simulink model "TosAeb"  (R2021a)
settings: Solver=FixedStepDiscrete  StartTime=0.0  StopTime=inf  FixedStep=0.01  RelTol=1e-3
undefined symbols (not defined in the file or in its folder): ACCModSts, AccSts1, AEB_ObjectMovingOrientation, AEB_ObjectMovingState, CN_BTCActive, CN_CurrentGearR, CN_CurrentGearR_DHT, CN_PTCActive, CN_VDCActive, CN_VSC_EspOff, CN_VSC_FCW_AEB_Error, CN_VSC_FCW_AEB_NoDispay, CN_VSC_FCW_AEB_PerfmDgdtn, CN_VSC_FCW_AEB_TempUnAvlbl, CN_VSC_VehStandstill, CNaccelAvoidMax, CNaccelAvoidMin, CNbrakePedalPress, CNcomfortStrLeftAccelMax, CNcomfortStrLeftAccelMin, CNcomfortStrRightAccelMax, CNcomfortStrRightAccelMin, CNDrvDoorOpen, CNDrvModeEco, CNDrvModeNorm, CNDrvModeOther, CNDrvModeSport, CNDrvSBRreminder, CNNull, CNobjInvalidID, CNPassDoorOpen, CNPassSBRreminder, CNPEBSAccelNegInf, CNPEBSAccelNull, CNPEBSTimeInf, CNPEBSTimeNull, CNSTOPMAX, CNSTOPNULL, CNsubjWidth, CNSwtOFF, CNSwtON, CNTTCMAX, CNTTCNULL, CNVehConfigAEBCycNone, CNVehConfigAEBNone, CNVehConfigAEBPedNone, CNVehConfigFCWNone, CNVehConfigJANone, CollisionSeverity, FALSE, FCWSnvty, JACycObjCalcInfoDefaultParams, JAPedObjCalcInfoDefaultParams, JAVehObjCalcInfoDefaultParams, latCycObjCalcInfoDefaultParams, latPedObjCalcInfoDefaultParams, longObjCalcInfoDefaultParams, ModSts, movingStatus, ObjectType ... (+1112)
libraries: simulink x3031, AEBSFunctionLib x50, Ta_Library x33, AEBSStateMachineLib x23, Mylibrary x12, simulink_extras x7, ObjSelect_lib x3, dspstat3 x2
(part 72 of 102)

== system TosAeb/FcwAebJa_Step_sys/ALG_AEBSystem/ALG_AEBController/AEB_LatObjInfoCalcApp/LatPedObjCalc/Subsystem/latPedObjInfoCalc/ObjCrossFlgCal/PedPreCollisionCalcFunc/SubjBoundingBox/SubjLLCoordinate ==
  inputSignals : Inport
  Add2 : Sum  Inputs=+--  OutDataTypeStr=single
  Add3 : Sum  Inputs=+-+  OutDataTypeStr=single
  Bus Selector2 : BusSelector  OutputSignals=dxPreRefPoint,dxRearBumperToRearAxis,sincosCoef.cosCoef,subjWidth,sincosCoef.sinCoef
  Bus Selector3 : BusSelector  OutputSignals=dyPreRefPoint,dxRearBumperToRearAxis,sincosCoef.sinCoef,subjWidth,sincosCoef.cosCoef
  Gain2 : Gain  Gain=0.5  OutDataTypeStr=single
  Gain3 : Gain  Gain=0.5  OutDataTypeStr=single
  Product{4..7} (4 alike) : Product  OutDataTypeStr=single
  dxPreLL : Outport
  dyPreLL : Outport  Port=2
  wires:
  Gain3/1 -> Add3/3
  Bus Selector3/3 -> Product6/2  "<sinCoef>"
  Add3/1 -> dyPreLL/1
  Product7/1 -> Gain3/1
  Product6/1 -> Add3/2
  Product4/1 -> Add2/2
  Bus Selector3/5 -> Product7/2  "<cosCoef>"
  Product5/1 -> Gain2/1
  Gain2/1 -> Add2/3
  Add2/1 -> dxPreLL/1
  Bus Selector3/4 -> Product7/1  "<subjWidth>"
  Bus Selector3/2 -> Product6/1  "<dxRearBumperToRearAxis>"
  Bus Selector3/1 -> Add3/1  "<dyPreRefPoint>"
  inputSignals/1 -> Bus Selector3/1, Bus Selector2/1
  Bus Selector2/4 -> Product5/1  "<subjWidth>"
  Bus Selector2/2 -> Product4/1  "<dxRearBumperToRearAxis>"
  Bus Selector2/3 -> Product4/2  "<cosCoef>"
  Bus Selector2/1 -> Add2/1  "<dxPreRefPoint>"
  Bus Selector2/5 -> Product5/2  "<sinCoef>"

== system TosAeb/FcwAebJa_Step_sys/ALG_AEBSystem/ALG_AEBController/AEB_LatObjInfoCalcApp/LatPedObjCalc/Subsystem/latPedObjInfoCalc/ObjCrossFlgCal/PedPreCollisionCalcFunc/SubjBoundingBox/SubjLUCoordinate ==
  inputSignals : Inport
  Add : Sum  Inputs=++-  OutDataTypeStr=single
  Add1 : Sum  Inputs=+++  OutDataTypeStr=single
  Bus Selector : BusSelector  OutputSignals=dxPreRefPoint,dxFrontBumperToRearAxis,sincosCoef.cosCoef,subjWidth,sincosCoef.sinCoef
  Bus Selector1 : BusSelector  OutputSignals=dyPreRefPoint,dxFrontBumperToRearAxis,sincosCoef.sinCoef,subjWidth,sincosCoef.cosCoef
  Gain : Gain  Gain=0.5  OutDataTypeStr=single
  Gain1 : Gain  Gain=0.5  OutDataTypeStr=single
  Product{,1..3} (4 alike) : Product  OutDataTypeStr=single
  dxPreLU : Outport
  dyPreLU : Outport  Port=2
  wires:
  Bus Selector/4 -> Product1/1  "<subjWidth>"
  Product3/1 -> Gain1/1
  Add/1 -> dxPreLU/1
  Bus Selector1/1 -> Add1/1  "<dyPreRefPoint>"
  Gain1/1 -> Add1/3
  Bus Selector1/4 -> Product3/1  "<subjWidth>"
  Product2/1 -> Add1/2
  Bus Selector/3 -> Product/2  "<cosCoef>"
  Add1/1 -> dyPreLU/1
  Bus Selector/5 -> Product1/2  "<sinCoef>"
  Bus Selector/2 -> Product/1  "<dxFrontBumperToRearAxis>"
  Bus Selector1/2 -> Product2/1  "<dxFrontBumperToRearAxis>"
  inputSignals/1 -> Bus Selector1/1, Bus Selector/1
  Bus Selector1/5 -> Product3/2  "<cosCoef>"
  Bus Selector/1 -> Add/1  "<dxPreRefPoint>"
  Gain/1 -> Add/3
  Product1/1 -> Gain/1
  Product/1 -> Add/2
  Bus Selector1/3 -> Product2/2  "<sinCoef>"

== system TosAeb/FcwAebJa_Step_sys/ALG_AEBSystem/ALG_AEBController/AEB_LatObjInfoCalcApp/LatPedObjCalc/Subsystem/latPedObjInfoCalc/ObjCrossFlgCal/PedPreCollisionCalcFunc/SubjBoundingBox/SubjRLCoordinate ==
  inputSignals : Inport
  Add6 : Sum  Inputs=+-+  OutDataTypeStr=single
  Add7 : Sum  Inputs=+--  OutDataTypeStr=single
  Bus Selector6 : BusSelector  OutputSignals=dxPreRefPoint,dxRearBumperToRearAxis,sincosCoef.cosCoef,subjWidth,sincosCoef.sinCoef
  Bus Selector7 : BusSelector  OutputSignals=dyPreRefPoint,dxRearBumperToRearAxis,sincosCoef.sinCoef,subjWidth,sincosCoef.cosCoef
  Gain6 : Gain  Gain=0.5  OutDataTypeStr=single
  Gain7 : Gain  Gain=0.5  OutDataTypeStr=single
  Product{12..15} (4 alike) : Product  OutDataTypeStr=single
  dxPreRL : Outport
  dyPreRL : Outport  Port=2
  wires:
  Product12/1 -> Add6/2
  Gain6/1 -> Add6/3
  Product13/1 -> Gain6/1
  Add6/1 -> dxPreRL/1
  Bus Selector6/4 -> Product13/1  "<subjWidth>"
  Bus Selector6/5 -> Product13/2  "<sinCoef>"
  Bus Selector6/1 -> Add6/1  "<dxPreRefPoint>"
  Bus Selector6/2 -> Product12/1  "<dxRearBumperToRearAxis>"
  Bus Selector6/3 -> Product12/2  "<cosCoef>"
  inputSignals/1 -> Bus Selector7/1, Bus Selector6/1
  Add7/1 -> dyPreRL/1
  Product14/1 -> Add7/2
  Gain7/1 -> Add7/3
  Bus Selector7/1 -> Add7/1  "<dyPreRefPoint>"
  Bus Selector7/2 -> Product14/1  "<dxRearBumperToRearAxis>"
  Bus Selector7/4 -> Product15/1  "<subjWidth>"
  Product15/1 -> Gain7/1
  Bus Selector7/5 -> Product15/2  "<cosCoef>"
  Bus Selector7/3 -> Product14/2  "<sinCoef>"

== system TosAeb/FcwAebJa_Step_sys/ALG_AEBSystem/ALG_AEBController/AEB_LatObjInfoCalcApp/LatPedObjCalc/Subsystem/latPedObjInfoCalc/ObjCrossFlgCal/PedPreCollisionCalcFunc/SubjBoundingBox/SubjRUCoordinate ==
  inputSignals : Inport
  Add4 : Sum  Inputs=+++  OutDataTypeStr=single
  Add5 : Sum  Inputs=++-  OutDataTypeStr=single
  Bus Selector4 : BusSelector  OutputSignals=dxPreRefPoint,dxFrontBumperToRearAxis,sincosCoef.cosCoef,subjWidth,sincosCoef.sinCoef
  Bus Selector5 : BusSelector  OutputSignals=dyPreRefPoint,dxFrontBumperToRearAxis,sincosCoef.sinCoef,subjWidth,sincosCoef.cosCoef
  Gain4 : Gain  Gain=0.5  OutDataTypeStr=single
  Gain5 : Gain  Gain=0.5  OutDataTypeStr=single
  Product{8..11} (4 alike) : Product  OutDataTypeStr=single
  dxPreRU : Outport
  dyPreRU : Outport  Port=2
  wires:
  Bus Selector4/4 -> Product9/1  "<subjWidth>"
  Bus Selector4/5 -> Product9/2  "<sinCoef>"
  Bus Selector4/3 -> Product8/2  "<cosCoef>"
  Bus Selector4/1 -> Add4/1  "<dxPreRefPoint>"
  inputSignals/1 -> Bus Selector5/1, Bus Selector4/1
  Bus Selector4/2 -> Product8/1  "<dxFrontBumperToRearAxis>"
  Product9/1 -> Gain4/1
  Bus Selector5/4 -> Product11/1  "<subjWidth>"
  Product10/1 -> Add5/2
  Product11/1 -> Gain5/1
  Add5/1 -> dyPreRU/1
  Gain5/1 -> Add5/3
  Bus Selector5/2 -> Product10/1  "<dxFrontBumperToRearAxis>"
  Bus Selector5/3 -> Product10/2  "<sinCoef>"
  Bus Selector5/5 -> Product11/2  "<cosCoef>"
  Add4/1 -> dxPreRU/1
  Gain4/1 -> Add4/3
  Product8/1 -> Add4/2
  Bus Selector5/1 -> Add5/1  "<dyPreRefPoint>"

== system TosAeb/FcwAebJa_Step_sys/ALG_AEBSystem/ALG_AEBController/AEB_LongTgtInfoCalc/LongObjCalcInfoCalc/AEBS_LongCollisionAssesApp/LatCollisionAvoidCalc/AccelLatAvoidCalc4SO/accelLatAvoidLeftCalc/CalculateAccelSteeringLeft/CalculateAccelSteeringLeft  ==
  dxRelTargetObjCalc : Inport
  dyRelTargetObjCalc : Inport  Port=2
  subjVel : Inport  Port=3
  Function : TriggerPort  TriggerType=function-call  VariantControl=Variant  PortDimensions=1
  Add : Sum
  Add1 : Sum
  Divide : Product  Inputs=*/
  Divide1 : Product  Inputs=*/
  Gain : Gain  Gain=2
  Math Function{,1..3} (4 alike) : Math  Operator=square  SignedPower=on  SaturateOnIntegerOverflow=on
  P_LongCA_LatAvoidLatDistMinSO : Constant  Value=P_AEB_LongCA_LatAvoidLatDistMinSO
  Subtract : Sum  Inputs=+-
  accelLatAvoidLeft : Outport
  wires:
  Math Function/1 -> Add/1
  Math Function2/1 -> Add/2
  dyRelTargetObjCalc/1 -> Math Function2/1, Add1/2
  P_LongCA_LatAvoidLatDistMinSO/1 -> Add1/1, Math Function1/1
  Add/1 -> Subtract/1
  Math Function1/1 -> Subtract/2
  dxRelTargetObjCalc/1 -> Math Function/1
  Add1/1 -> Gain/1
  Subtract/1 -> Divide/1
  Gain/1 -> Divide/2
  Divide/1 -> Divide1/2
  Divide1/1 -> accelLatAvoidLeft/1
  subjVel/1 -> Math Function3/1
  Math Function3/1 -> Divide1/1

== system TosAeb/FcwAebJa_Step_sys/ALG_AEBSystem/ALG_AEBController/AEB_LongTgtInfoCalc/LongObjCalcInfoCalc/AEBS_LongCollisionAssesApp/LatCollisionAvoidCalc/AccelLatAvoidCalc4SO/accelLatAvoidLeftCalc/CalculateAccelSteeringLeft/tempFlgCalc ==
  dxRelTargetObjCalc : Inport
  dyRelTargetObjCalc : Inport  Port=2
  Add : Sum
  Math Function : Math  Operator=square  SignedPower=on  SaturateOnIntegerOverflow=on
  Math Function1 : Math  Operator=square  SignedPower=on  SaturateOnIntegerOverflow=on
  Math Function2 : Math  Operator=square  SignedPower=on  SaturateOnIntegerOverflow=on
  Relational Operator : RelationalOperator  Operator=<  OutDataTypeStr=boolean  RndMeth=Simplest
  dyMinStationary : Constant  Value=PcomfortStrLatDistMinSO
  tempFlg : Outport
  wires:
  Relational Operator/1 -> tempFlg/1
  Math Function/1 -> Add/1
  Math Function1/1 -> Relational Operator/1
  Add/1 -> Relational Operator/2
  Math Function2/1 -> Add/2
  dyMinStationary/1 -> Math Function1/1
  dyRelTargetObjCalc/1 -> Math Function2/1
  dxRelTargetObjCalc/1 -> Math Function/1

== system TosAeb/FcwAebJa_Step_sys/ALG_AEBSystem/ALG_AEBController/AEB_LongTgtInfoCalc/LongObjCalcInfoCalc/AEBS_LongCollisionAssesApp/LatCollisionAvoidCalc/AccelLatAvoidCalc4SO/accelLatAvoidRightCalc/CalculateAccelSteeringRight/CalculateAccelSteeringLeft  ==
  note: NOTE:
      | accelSteeringRight < 0
  dxRelTargetObjCalc : Inport
  dyRelTargetObjCalc : Inport  Port=2
  subjVel : Inport  Port=3
  Function : TriggerPort  TriggerType=function-call  VariantControl=Variant  PortDimensions=1
  Add : Sum
  Add1 : Sum  Inputs=+-
  Divide : Product  Inputs=*/
  Divide1 : Product  Inputs=*/
  Gain : Gain  Gain=2
  Gain1 : Gain  Gain=-1
  Math Function{,1..3} (4 alike) : Math  Operator=square  SignedPower=on  SaturateOnIntegerOverflow=on
  Subtract : Sum  Inputs=+-
  dyMin : Constant  Value=PcomfortStrLatDistMinSO
  accelLatAvoidRight : Outport
  wires:
  Gain1/1 -> accelLatAvoidRight/1
  Math Function/1 -> Add/1
  Math Function2/1 -> Add/2
  dyRelTargetObjCalc/1 -> Math Function2/1, Add1/2
  dyMin/1 -> Add1/1, Math Function1/1
  Add/1 -> Subtract/1
  Math Function1/1 -> Subtract/2
  dxRelTargetObjCalc/1 -> Math Function/1
  Add1/1 -> Gain/1
  Subtract/1 -> Divide/1
  Gain/1 -> Divide/2
  Divide/1 -> Divide1/2
  Divide1/1 -> Gain1/1
  subjVel/1 -> Math Function3/1
  Math Function3/1 -> Divide1/1

== system TosAeb/FcwAebJa_Step_sys/ALG_AEBSystem/ALG_AEBController/AEB_LongTgtInfoCalc/LongObjCalcInfoCalc/AEBS_LongCollisionAssesApp/LatCollisionAvoidCalc/AccelLatAvoidCalc4SO/accelLatAvoidRightCalc/CalculateAccelSteeringRight/tempFlgCalc ==
  dxRelTargetObjCalc : Inport
  dyRelTargetObjCalc : Inport  Port=2
  Add : Sum
  Math Function : Math  Operator=square  SignedPower=on  SaturateOnIntegerOverflow=on
  Math Function1 : Math  Operator=square  SignedPower=on  SaturateOnIntegerOverflow=on
  Math Function2 : Math  Operator=square  SignedPower=on  SaturateOnIntegerOverflow=on
  Relational Operator : RelationalOperator  Operator=<  OutDataTypeStr=boolean  RndMeth=Simplest
  dyMinStationary : Constant  Value=PcomfortStrLatDistMinSO
  tempFlg : Outport
  wires:
  Relational Operator/1 -> tempFlg/1
  Math Function/1 -> Add/1
  Math Function1/1 -> Relational Operator/1
  Add/1 -> Relational Operator/2
  Math Function2/1 -> Add/2
  dyMinStationary/1 -> Math Function1/1
  dyRelTargetObjCalc/1 -> Math Function2/1
  dxRelTargetObjCalc/1 -> Math Function/1

== system TosAeb/FcwAebJa_Step_sys/ALG_AEBSystem/ALG_AEBController/AEB_LongTgtInfoCalc/LongObjCalcInfoCalc/AEBS_LongCollisionAssesApp/LatCollisionAvoidCalc/AccelLatAvoidCalcMO/MovingObjLatCollisionAvoidCalc/Condition2/TTCIsZero ==
  TTC : Inport
  Compare To Constant : simulink/Logic and Bit Operations/Compare To Constant  relop=<  const=0.1  OutDataTypeStr=boolean
  TTCIsZeroFlg : Outport
  wires:
  Compare To Constant/1 -> TTCIsZeroFlg/1
  TTC/1 -> Compare To Constant/1

== system TosAeb/FcwAebJa_Step_sys/ALG_AEBSystem/ALG_AEBController/AEB_LongTgtInfoCalc/LongObjCalcInfoCalc/AEBS_LongCollisionAssesApp/LatCollisionAvoidCalc/AccelLatAvoidCalcMO/MovingObjLatCollisionAvoidCalc/Condition2/latAvoidAccelMO ==
  dy : Inport
  vy : Inport  Port=2
  TTC : Inport  Port=3
  Action Port : ActionPort  ActionPortLabel=else
  Bus Creator : BusCreator  Inputs=2
  Divide : Product  Inputs=*/
  Divide1 : Product  Inputs=*/
  Gain : Gain  Gain=-2
  Gain1 : Gain  Gain=-2
  Math Function : Math  Operator=square  SignedPower=on  SaturateOnIntegerOverflow=on
  Math Function1 : Math  Operator=square  SignedPower=on  SaturateOnIntegerOverflow=on
  P_LongCA_LatAvoidLatDistMinSO : Constant  Value=P_AEB_LongCA_LatAvoidLatDistMinSO
  P_LongCA_LatAvoidLatDistMinSO1 : Constant  Value=P_AEB_LongCA_LatAvoidLatDistMinSO
  Product : Product
  Product1 : Product
  Saturation : Saturate  UpperLimit=CNcomfortStrRightAccelMax  LowerLimit=CNcomfortStrRightAccelMin
      settings: AttributesFormatString=|
      | UpperLimit:%<UpperLimit>
      | LowerLimit:%<LowerLimit>
  Saturation1 : Saturate  UpperLimit=CNcomfortStrLeftAccelMax  LowerLimit=CNcomfortStrLeftAccelMin
      settings: AttributesFormatString=|
      | UpperLimit:%<UpperLimit>
      | LowerLimit:%<LowerLimit>
  Subtract : Sum  Inputs=+--
  Subtract1 : Sum  Inputs=---
  accelLatAvoid : Outport
  wires:
  Gain1/1 -> Divide/1
  Gain/1 -> Divide1/1
  Divide/1 -> Saturation/1  "comfortStrAccelRightUnLimited"
  Divide1/1 -> Saturation1/1  "comfortStrAccelLeftUnLimited"
  Bus Creator/1 -> accelLatAvoid/1
  vy/1 -> [vyRelTargetObjCalc], Product1/1
  TTC/1 -> Product/2, Math Function/1, Product1/2, Math Function1/1
  Product1/1 -> Subtract1/3
  P_LongCA_LatAvoidLatDistMinSO/1 -> Subtract1/1
  dy/1 -> [dyRelTargetObjCalc], Subtract1/2
  Math Function1/1 -> Divide1/2
  Subtract1/1 -> Gain/1
  Subtract/1 -> Gain1/1
  Math Function/1 -> Divide/2
  [dyRelTargetObjCalc] -> Subtract/2
  P_LongCA_LatAvoidLatDistMinSO1/1 -> Subtract/1
  Product/1 -> Subtract/3
  [vyRelTargetObjCalc] -> Product/1
  Saturation/1 -> Bus Creator/2  "accelLatAvoidRight"
  Saturation1/1 -> Bus Creator/1  "accelLatAvoidLeft"

== system TosAeb/FcwAebJa_Step_sys/ALG_AEBSystem/ALG_AEBController/AEB_LongTgtInfoCalc/LongObjCalcInfoCalc/AEBS_LongCollisionAssesApp/LatCollisionAvoidCalc/AccelLatAvoidCalcMO/MovingObjLatCollisionAvoidCalc/Condition2/latAvoidAccelMoMax ==
  Action Port : ActionPort  ActionPortLabel=if(u1)
  Bus Creator : BusCreator  Inputs=2
  Constant : Constant  Value=CNcomfortStrLeftAccelMax
  Constant1 : Constant  Value=CNcomfortStrRightAccelMin
  accelLatAvoid : Outport
  wires:
  Constant1/1 -> Bus Creator/2  "accelLatAvoidRight"
  Bus Creator/1 -> accelLatAvoid/1
  Constant/1 -> Bus Creator/1  "accelLatAvoidLeft"

== system TosAeb/FcwAebJa_Step_sys/ALG_AEBSystem/ALG_AEBController/AEB_LongTgtInfoCalc/LongObjCalcInfoCalc/AEBS_LongCollisionAssesApp/LongCollisionAvoidAssess/HighLvlBrkCalc/HighLvlBrkCalc4Brake/accelAvoidHighLvlBrkSituation1/accelAvoidLowLvlBrkCalc ==
  vx : Inport
  axAbsObj : Inport  Port=2
  vxAbsObj : Inport  Port=3
  subjVel : Inport  Port=4
  tObjStop : Inport  Port=5
  dxEgoBrake : Inport  Port=6
  AccelAvoidSituationJudge : Stateflow chart  TreatAsAtomicUnit=on
  Bus Creator : BusCreator  Inputs=6
  Bus Selector : BusSelector  OutputSignals=vx,axAbsObj
  Bus Selector1 : BusSelector  OutputSignals=dxEgoBrake,vx,tObjStop,subjVel,vxAbsObj,axAbsObj
  Bus Selector2 : BusSelector  OutputSignals=axAbsObj,vx,dxEgoBrake
  Bus Selector3 : BusSelector  OutputSignals=subjVel,vxAbsObj,axAbsObj,dxEgoBrake
  Merge : Merge  Inputs=4
  Situation1 : Subsystem  TreatAsAtomicUnit=on
  Situation2 : Subsystem  TreatAsAtomicUnit=on
  Situation3 : Subsystem  TreatAsAtomicUnit=on
  Situation4 : Subsystem  TreatAsAtomicUnit=on
  accelAvoidLowLvlBrkLimit : Subsystem
  accelAvoidLowLvlBrk : Outport
  wires:
  Bus Selector1/6 -> Situation1/6(axAbsObj)  "<axAbsObj>"
  Bus Selector1/5 -> Situation1/5(vxAbsObj)  "<vxAbsObj>"
  Bus Selector1/4 -> Situation1/4(subjVel)  "<subjVel>"
  Bus Selector1/3 -> Situation1/3(tObjStop)  "<tObjStop>"
  Bus Selector1/2 -> Situation1/2(vx)  "<vx>"
  Bus Selector1/1 -> Situation1/1(dxEgoBrake)  "<dxEgoBrake>"
  AccelAvoidSituationJudge/1(Situation1()) -> Situation1/Trigger
  Situation1/1(accelAvoidLowLvlBrk) -> Merge/1
  Situation2/1(accelAvoidLowLvlBrk) -> Merge/2
  Situation3/1(accelAvoidLowLvlBrk) -> Merge/3
  Situation4/1(accelAvoidLowLvlBrk) -> Merge/4
  AccelAvoidSituationJudge/2(Situation2()) -> [Situation2]
  AccelAvoidSituationJudge/3(Situation3()) -> [Situation3]
  AccelAvoidSituationJudge/4(Situation4()) -> [Situation4]
  [Situation2] -> Situation2/Trigger
  [Situation3] -> Situation3/Trigger
  [Situation4] -> Situation4/Trigger
  vxAbsObj/1 -> Bus Creator/3  "vxAbsObj"
  subjVel/1 -> Bus Creator/4  "subjVel"
  dxEgoBrake/1 -> Bus Creator/6  "dxEgoBrake"
  vx/1 -> Bus Creator/1  "vx"
  axAbsObj/1 -> Bus Creator/2  "axAbsObj"
  tObjStop/1 -> Bus Creator/5  "tObjStop"
  Bus Creator/1 -> Bus Selector3/1, Bus Selector2/1, Bus Selector1/1, Bus Selector/1
  Bus Selector/1 -> AccelAvoidSituationJudge/1(vx)  "<vx>"
  Bus Selector/2 -> AccelAvoidSituationJudge/2(axAbsObj)  "<axAbsObj>"
  Bus Selector2/3 -> Situation2/3(dxEgoBrake)  "<dxEgoBrake>"
  Bus Selector2/2 -> Situation2/2(vx)  "<vx>"
  Bus Selector2/1 -> Situation2/1(axAbsObj)  "<axAbsObj>"
  Bus Selector3/1 -> Situation3/1(subjVel)  "<subjVel>"
  Bus Selector3/2 -> Situation3/2(vxAbsObj)  "<vxAbsObj>"
  Bus Selector3/3 -> Situation3/3(axAbsObj)  "<axAbsObj>"
  Bus Selector3/4 -> Situation3/4(dxEgoBrake)  "<dxEgoBrake>"
  Merge/1 -> accelAvoidLowLvlBrkLimit/1(accelAvoidLowLvlBrkNoLimit)
  accelAvoidLowLvlBrkLimit/1(accelAvoidLowLvlBrk) -> accelAvoidLowLvlBrk/1

== system TosAeb/FcwAebJa_Step_sys/ALG_AEBSystem/ALG_AEBController/AEB_LongTgtInfoCalc/LongObjCalcInfoCalc/AEBS_LongCollisionAssesApp/LongCollisionAvoidAssess/HighLvlBrkCalc/HighLvlBrkCalc4Brake/accelAvoidHighLvlBrkSituation1/accelAvoidLowLvlBrkCalc2 ==
  vx : Inport
  axAbsObj : Inport  Port=2
  vxAbsObj : Inport  Port=3
  subjVel : Inport  Port=4
  tObjStop : Inport  Port=5
  dxEgoBrake : Inport  Port=6
  AccelAvoidSituationJudge : Stateflow chart  TreatAsAtomicUnit=on
  Bus Creator : BusCreator  Inputs=6
  Bus Selector : BusSelector  OutputSignals=vxRelTargetObjCalc,axAbsTargetObjCalc
  Bus Selector1 : BusSelector  OutputSignals=dxEgoBrake,vxRelTargetObjCalc,tObjStop,subjVel,vxAbsTargetObjCalc,axAbsTargetObjCalc
  Bus Selector2 : BusSelector  OutputSignals=axAbsTargetObjCalc,vxRelTargetObjCalc,dxEgoBrake
  Bus Selector3 : BusSelector  OutputSignals=subjVel,vxAbsTargetObjCalc,axAbsTargetObjCalc,dxEgoBrake
  Merge : Merge  Inputs=4
  Situation1 : Subsystem  TreatAsAtomicUnit=on
  Situation2 : Subsystem  TreatAsAtomicUnit=on
  Situation3 : Subsystem  TreatAsAtomicUnit=on
  Situation4 : Subsystem  TreatAsAtomicUnit=on
  accelAvoidLowLvlBrkLimit : Subsystem
  accelAvoidLowLvlBrk : Outport
  wires:
  accelAvoidLowLvlBrkLimit/1(accelAvoidLowLvlBrk) -> accelAvoidLowLvlBrk/1
  Merge/1 -> accelAvoidLowLvlBrkLimit/1(accelAvoidLowLvlBrkNoLimit)
  Bus Selector3/4 -> Situation3/4(dxEgoBrake)  "<dxEgoBrake>"
  Bus Selector3/3 -> Situation3/3(axAbsObj)  "<axAbsTargetObjCalc>"
  Bus Selector3/2 -> Situation3/2(vxAbsObj)  "<vxAbsTargetObjCalc>"
  Bus Selector3/1 -> Situation3/1(subjVel)  "<subjVel>"
  Bus Selector2/1 -> Situation2/1(axAbsObj)  "<axAbsTargetObjCalc>"
  Bus Selector2/2 -> Situation2/2(vx)  "<vxRelTargetObjCalc>"
  Bus Selector2/3 -> Situation2/3(dxEgoBrake)  "<dxEgoBrake>"
  Bus Selector/2 -> AccelAvoidSituationJudge/2(axAbsTargetObjCalc)  "<axAbsTargetObjCalc>"
  Bus Selector/1 -> AccelAvoidSituationJudge/1(vxRelTargetObjCalc)  "<vxRelTargetObjCalc>"
  Bus Creator/1 -> Bus Selector/1, Bus Selector1/1, Bus Selector2/1, Bus Selector3/1
  tObjStop/1 -> Bus Creator/5  "tObjStop"
  axAbsObj/1 -> Bus Creator/2  "axAbsTargetObjCalc"
  vx/1 -> Bus Creator/1  "vxRelTargetObjCalc"
  dxEgoBrake/1 -> Bus Creator/6  "dxEgoBrake"
  subjVel/1 -> Bus Creator/4  "subjVel"
  vxAbsObj/1 -> Bus Creator/3  "vxAbsTargetObjCalc"
  [Situation4] -> Situation4/Trigger
  [Situation3] -> Situation3/Trigger
  [Situation2] -> Situation2/Trigger
  AccelAvoidSituationJudge/4(Situation4()) -> [Situation4]
  AccelAvoidSituationJudge/3(Situation3()) -> [Situation3]
  AccelAvoidSituationJudge/2(Situation2()) -> [Situation2]
  Situation4/1(accelAvoidLowLvlBrk) -> Merge/4
  Situation3/1(accelAvoidLowLvlBrk) -> Merge/3
  Situation2/1(accelAvoidLowLvlBrk) -> Merge/2
  Situation1/1(accelAvoidLowLvlBrk) -> Merge/1
  AccelAvoidSituationJudge/1(Situation1()) -> Situation1/Trigger
  Bus Selector1/1 -> Situation1/1(dxEgoBrake)  "<dxEgoBrake>"
  Bus Selector1/2 -> Situation1/2(vx)  "<vxRelTargetObjCalc>"
  Bus Selector1/3 -> Situation1/3(tObjStop)  "<tObjStop>"
  Bus Selector1/4 -> Situation1/4(subjVel)  "<subjVel>"
  Bus Selector1/5 -> Situation1/5(vxAbsObj)  "<vxAbsTargetObjCalc>"
  Bus Selector1/6 -> Situation1/6(axAbsObj)  "<axAbsTargetObjCalc>"

== system TosAeb/FcwAebJa_Step_sys/ALG_AEBSystem/ALG_AEBController/AEB_LongTgtInfoCalc/LongObjCalcInfoCalc/AEBS_LongCollisionAssesApp/LongCollisionAvoidAssess/HighLvlBrkCalc/HighLvlBrkCalc4Brake/accelAvoidHighLvlBrkSituation2/CollisionJuge ==
  tReaction : Inport
  TTC : Inport  Port=2
  Relational Operator : RelationalOperator  Operator=>  OutDataTypeStr=boolean  RndMeth=Simplest
  collisonFlg : Outport
  wires:
  TTC/1 -> Relational Operator/2
  Relational Operator/1 -> collisonFlg/1
  tReaction/1 -> Relational Operator/1

== system TosAeb/FcwAebJa_Step_sys/ALG_AEBSystem/ALG_AEBController/AEB_LongTgtInfoCalc/LongObjCalcInfoCalc/AEBS_LongCollisionAssesApp/LongCollisionAvoidAssess/HighLvlBrkCalc/HighLvlBrkCalc4Brake/accelAvoidHighLvlBrkSituation2/CollisonOcurred ==
  Action Port : ActionPort  ActionPortLabel=if(u1)
  Constant : Constant  Value=CNPEBSAccelNegInf
  accelAvoidHighLvlBrk : Outport
  wires:
  Constant/1 -> accelAvoidHighLvlBrk/1  "CNPEBSAccelNegInf"

== system TosAeb/FcwAebJa_Step_sys/ALG_AEBSystem/ALG_AEBController/AEB_LongTgtInfoCalc/LongObjCalcInfoCalc/AEBS_LongCollisionAssesApp/LongCollisionAvoidAssess/HighLvlBrkCalc/HighLvlBrkCalc4Brake/accelAvoidHighLvlBrkSituation2/accelAvoidHighLvlBrkCalc ==
  dxFrontBumper : Inport
  vx : Inport  Port=2
  tReaction : Inport  Port=3
  ax : Inport  Port=4
  subjLongAccel : Inport  Port=5
  subjVel : Inport  Port=6
  axAbsObj : Inport  Port=7
  Action Port : ActionPort  ActionPortLabel=else
  If : If  IfExpression=u1 <= 0
  If Action Subsystem : Subsystem  TreatAsAtomicUnit=on
  If Action Subsystem1 : Subsystem  TreatAsAtomicUnit=on
  Merge : Merge
  MovingSateCalcAfterReactionTime : Subsystem
  tObjStopCalc : Subsystem  TreatAsAtomicUnit=on
  accelAvoidHighLvlBrk : Outport
  wires:
  tObjStopCalc/1(tStop) -> If Action Subsystem1/5(tObjStop)
  Merge/1 -> accelAvoidHighLvlBrk/1
  vx/1 -> MovingSateCalcAfterReactionTime/2(vx)
  tReaction/1 -> MovingSateCalcAfterReactionTime/3(tReaction)
  ax/1 -> MovingSateCalcAfterReactionTime/4(ax)
  subjVel/1 -> MovingSateCalcAfterReactionTime/6(subjVel)
  subjLongAccel/1 -> MovingSateCalcAfterReactionTime/5(subjLongAccel)
  MovingSateCalcAfterReactionTime/1(dxFrontBumperPredict) -> [dxRelTargetPredict], If/1
  If/1 -> If Action Subsystem/Action
  If/2 -> If Action Subsystem1/Action
  If Action Subsystem1/1(accelAvoidHighLvlBrk) -> Merge/2
  If Action Subsystem/1(accelAvoidHighLvlBrk) -> Merge/1
  MovingSateCalcAfterReactionTime/3(vxAbsObjPredict) -> tObjStopCalc/2(velRel), If Action Subsystem1/3(vxAbsObj)
  axAbsObj/1 -> tObjStopCalc/1(accelRel), If Action Subsystem1/2(axAbsObj)
  MovingSateCalcAfterReactionTime/2(vxPredict) -> If Action Subsystem1/1(vx)
  MovingSateCalcAfterReactionTime/4(subjVelPredict) -> [subjVelPredict]
  [subjVelPredict] -> If Action Subsystem1/4(subjVel)
  [dxRelTargetPredict] -> If Action Subsystem1/6(dxEgoBrake)
  dxFrontBumper/1 -> MovingSateCalcAfterReactionTime/1(dxFrontBumper)

== system TosAeb/FcwAebJa_Step_sys/ALG_AEBSystem/ALG_AEBController/AEB_LongTgtInfoCalc/LongObjCalcInfoCalc/AEBS_LongCollisionAssesApp/LongCollisionAvoidAssess/HighLvlBrkCalc/HighLvlBrkCalc4Brake/accelAvoidHighLvlBrkSituation3/CollisionJuge ==
  tReaction : Inport
  TTC : Inport  Port=2
  Relational Operator : RelationalOperator  Operator=>  OutDataTypeStr=boolean  RndMeth=Simplest
  collisonFlg : Outport
  wires:
  TTC/1 -> Relational Operator/2
  Relational Operator/1 -> collisonFlg/1
  tReaction/1 -> Relational Operator/1

== system TosAeb/FcwAebJa_Step_sys/ALG_AEBSystem/ALG_AEBController/AEB_LongTgtInfoCalc/LongObjCalcInfoCalc/AEBS_LongCollisionAssesApp/LongCollisionAvoidAssess/HighLvlBrkCalc/HighLvlBrkCalc4Brake/accelAvoidHighLvlBrkSituation3/If Action Subsystem ==
  Action Port : ActionPort  ActionPortLabel=if(u1)
  Constant : Constant  Value=CNaccelAvoidMin
  accelAvoidHighLvlBrk : Outport
  wires:
  Constant/1 -> accelAvoidHighLvlBrk/1  "CNPEBSAccelNegInf"

== system TosAeb/FcwAebJa_Step_sys/ALG_AEBSystem/ALG_AEBController/AEB_LongTgtInfoCalc/LongObjCalcInfoCalc/AEBS_LongCollisionAssesApp/LongCollisionAvoidAssess/HighLvlBrkCalc/HighLvlBrkCalc4Brake/accelAvoidHighLvlBrkSituation3/If Action Subsystem1 ==
  subjLongAccel : Inport
  tReaction : Inport  Port=2
  subjVel : Inport  Port=3
  axAbsObj : Inport  Port=4
  vxAbsObj : Inport  Port=5
  dxFrontBumper : Inport  Port=6
  Action Port : ActionPort  ActionPortLabel=else
  CollisionOcurredCase : Subsystem  TreatAsAtomicUnit=on
  DistReactionCalc : Subsystem
  If : If  IfExpression=u1
  Merge : Merge
  accelAvoidHighLvlBrkCalc : Subsystem  TreatAsAtomicUnit=on
  accelAvoidHighLvlBrk : Outport
  wires:
  subjLongAccel/1 -> [subjAccel], DistReactionCalc/1(subjLongAccel)
  tReaction/1 -> [tReaction], DistReactionCalc/2(tReaction)
  subjVel/1 -> [subjVel], DistReactionCalc/3(subjVel)
  axAbsObj/1 -> DistReactionCalc/4(axAbsObj)
  vxAbsObj/1 -> DistReactionCalc/5(vxAbsObj)
  Merge/1 -> accelAvoidHighLvlBrk/1
  dxFrontBumper/1 -> DistReactionCalc/6(dxFrontBumper)
  DistReactionCalc/1(collisionOcurredFlag) -> If/1
  If/1 -> CollisionOcurredCase/Action
  If/2 -> accelAvoidHighLvlBrkCalc/Action
  CollisionOcurredCase/1(accelAvoidHighLvlBrk) -> Merge/1
  DistReactionCalc/2(dxRelTargetPredict) -> accelAvoidHighLvlBrkCalc/4(dxEgoBrake)
  [subjAccel] -> accelAvoidHighLvlBrkCalc/1(subjLongAccel)
  [tReaction] -> accelAvoidHighLvlBrkCalc/2(tReaction)
  [subjVel] -> accelAvoidHighLvlBrkCalc/3(subjVel)
  accelAvoidHighLvlBrkCalc/1(accelAvoidHighLvlBrk) -> Merge/2

== system TosAeb/FcwAebJa_Step_sys/ALG_AEBSystem/ALG_AEBController/AEB_LongTgtInfoCalc/LongObjCalcInfoCalc/AEBS_LongCollisionAssesApp/LongCollisionAvoidAssess/HighLvlBrkCalc/HighLvlBrkCalc4Brake/accelAvoidHighLvlBrkSituation4/accelAvoidHighLvlBrkCalc ==
  tReaction : Inport
  TTC : Inport  Port=2
  Constant : Constant  Value=CNaccelAvoidMax
  Constant1 : Constant  Value=CNaccelAvoidMin
  Relational Operator : RelationalOperator  Operator=<=  OutDataTypeStr=boolean  RndMeth=Simplest
  Switch : Switch  Criteria=u2 ~= 0
  accelAvoidHighLvlBrk : Outport
  wires:
  tReaction/1 -> Relational Operator/1
  Relational Operator/1 -> Switch/2
  TTC/1 -> Relational Operator/2
  Constant/1 -> Switch/1
  Constant1/1 -> Switch/3
  Switch/1 -> accelAvoidHighLvlBrk/1

== system TosAeb/FcwAebJa_Step_sys/ALG_AEBSystem/ALG_AEBController/AEB_LongTgtInfoCalc/LongObjCalcInfoCalc/AEBS_LongCollisionAssesApp/LongCollisionAvoidAssess/HighLvlBrkCalc/HighLvlBrkCalc4Brake/accelAvoidHighLvlBrkSituation5/If Action Subsystem ==
  tReaction : Inport
  TTC : Inport  Port=2
  Action Port : ActionPort  ActionPortLabel=if(u1 <= u2)
  Constant : Constant  Value=CNaccelAvoidMax
  Constant1 : Constant  Value=CNaccelAvoidMin
  Relational Operator : RelationalOperator  Operator=<=  OutDataTypeStr=boolean  RndMeth=Simplest
  Switch : Switch  Criteria=u2 > Threshold
  accelAvoidHighLvlBrk : Outport
  wires:
  tReaction/1 -> Relational Operator/1
  TTC/1 -> Relational Operator/2
  Relational Operator/1 -> Switch/2
  Constant/1 -> Switch/1
  Constant1/1 -> Switch/3
  Switch/1 -> accelAvoidHighLvlBrk/1

== system TosAeb/FcwAebJa_Step_sys/ALG_AEBSystem/ALG_AEBController/AEB_LongTgtInfoCalc/LongObjCalcInfoCalc/AEBS_LongCollisionAssesApp/LongCollisionAvoidAssess/HighLvlBrkCalc/HighLvlBrkCalc4Brake/accelAvoidHighLvlBrkSituation5/If Action Subsystem1 ==
  tReaction : Inport
  TTC : Inport  Port=2
  subjLongAccel : Inport  Port=3
  tEgoStop : Inport  Port=4
  subjVel : Inport  Port=5
  axAbsObj : Inport  Port=6
  dxFrontBumper : Inport  Port=7
  vxAbsObj : Inport  Port=8
  Action Port : ActionPort  ActionPortLabel=else
  CollisionJuge : Subsystem
  If : If  IfExpression=u1
  If Action Subsystem : Subsystem  TreatAsAtomicUnit=on
  If Action Subsystem1 : Subsystem  TreatAsAtomicUnit=on
  Merge : Merge
  accelAvoidHighLvlBrk : Outport
  wires:
  tEgoStop/1 -> If Action Subsystem1/2(tEgoStop)
  CollisionJuge/1(collisonFlg) -> If/1
  TTC/1 -> CollisionJuge/2(TTC)
  If/1 -> If Action Subsystem/Action
  If/2 -> If Action Subsystem1/Action
  If Action Subsystem/1(accelAvoidHighLvlBrk) -> Merge/1
  If Action Subsystem1/1(accelAvoidHighLvlBrk) -> Merge/2
  Merge/1 -> accelAvoidHighLvlBrk/1
  tReaction/1 -> CollisionJuge/1(tReaction)
  subjLongAccel/1 -> If Action Subsystem1/1(subjLongAccel)
  subjVel/1 -> If Action Subsystem1/3(subjVel)
  axAbsObj/1 -> If Action Subsystem1/4(axAbsObj)
  vxAbsObj/1 -> If Action Subsystem1/5(vxAbsObj)
  dxFrontBumper/1 -> If Action Subsystem1/6(dxFrontBumper)

== system TosAeb/FcwAebJa_Step_sys/ALG_AEBSystem/ALG_AEBController/AEB_LongTgtInfoCalc/LongObjCalcInfoCalc/AEBS_LongCollisionAssesApp/LongCollisionAvoidAssess/HighLvlBrkCalc/HighLvlBrkCalc4Warning/accelAvoidHighLvlBrkSituation1/accelAvoidLowLvlBrkCalc ==
  vx : Inport
  axAbsObj : Inport  Port=2
  vxAbsObj : Inport  Port=3
  subjVel : Inport  Port=4
  tObjStop : Inport  Port=5
  dxEgoBrake : Inport  Port=6
  AccelAvoidSituationJudge : Stateflow chart  TreatAsAtomicUnit=on
  Bus Creator : BusCreator  Inputs=6
  Bus Selector : BusSelector  OutputSignals=vx,axAbsObj
  Bus Selector1 : BusSelector  OutputSignals=dxEgoBrake,vx,tObjStop,subjVel,vxAbsObj,axAbsObj
  Bus Selector2 : BusSelector  OutputSignals=axAbsObj,vx,dxEgoBrake
  Bus Selector3 : BusSelector  OutputSignals=subjVel,vxAbsObj,axAbsObj,dxEgoBrake
  Merge : Merge  Inputs=4
  Situation1 : Subsystem  TreatAsAtomicUnit=on
  Situation2 : Subsystem  TreatAsAtomicUnit=on
  Situation3 : Subsystem  TreatAsAtomicUnit=on
  Situation4 : Subsystem  TreatAsAtomicUnit=on
  accelAvoidLowLvlBrkLimit : Subsystem
  accelAvoidLowLvlBrk : Outport
  wires:
  accelAvoidLowLvlBrkLimit/1(accelAvoidLowLvlBrk) -> accelAvoidLowLvlBrk/1
  Merge/1 -> accelAvoidLowLvlBrkLimit/1(accelAvoidLowLvlBrkNoLimit)
  Bus Selector3/4 -> Situation3/4(dxEgoBrake)  "<dxEgoBrake>"
  Bus Selector3/3 -> Situation3/3(axAbsObj)  "<axAbsObj>"
  Bus Selector3/2 -> Situation3/2(vxAbsObj)  "<vxAbsObj>"
  Bus Selector3/1 -> Situation3/1(subjVel)  "<subjVel>"
  Bus Selector2/1 -> Situation2/1(axAbsObj)  "<axAbsObj>"
  Bus Selector2/2 -> Situation2/2(vx)  "<vx>"
  Bus Selector2/3 -> Situation2/3(dxEgoBrake)  "<dxEgoBrake>"
  Bus Selector/2 -> AccelAvoidSituationJudge/2(axAbsObj)  "<axAbsObj>"
  Bus Selector/1 -> AccelAvoidSituationJudge/1(vx)  "<vx>"
  Bus Creator/1 -> Bus Selector/1, Bus Selector1/1, Bus Selector2/1, Bus Selector3/1
  tObjStop/1 -> Bus Creator/5  "tObjStop"
  axAbsObj/1 -> Bus Creator/2  "axAbsObj"
  vx/1 -> Bus Creator/1  "vx"
  dxEgoBrake/1 -> Bus Creator/6  "dxEgoBrake"
  subjVel/1 -> Bus Creator/4  "subjVel"
  vxAbsObj/1 -> Bus Creator/3  "vxAbsObj"
  [Situation4] -> Situation4/Trigger
  [Situation3] -> Situation3/Trigger
  [Situation2] -> Situation2/Trigger
  AccelAvoidSituationJudge/4(Situation4()) -> [Situation4]
  AccelAvoidSituationJudge/3(Situation3()) -> [Situation3]
  AccelAvoidSituationJudge/2(Situation2()) -> [Situation2]
  Situation4/1(accelAvoidLowLvlBrk) -> Merge/4
  Situation3/1(accelAvoidLowLvlBrk) -> Merge/3
  Situation2/1(accelAvoidLowLvlBrk) -> Merge/2
  Situation1/1(accelAvoidLowLvlBrk) -> Merge/1
  AccelAvoidSituationJudge/1(Situation1()) -> Situation1/Trigger
  Bus Selector1/1 -> Situation1/1(dxEgoBrake)  "<dxEgoBrake>"
  Bus Selector1/2 -> Situation1/2(vx)  "<vx>"
  Bus Selector1/3 -> Situation1/3(tObjStop)  "<tObjStop>"
  Bus Selector1/4 -> Situation1/4(subjVel)  "<subjVel>"
  Bus Selector1/5 -> Situation1/5(vxAbsObj)  "<vxAbsObj>"
  Bus Selector1/6 -> Situation1/6(axAbsObj)  "<axAbsObj>"

== system TosAeb/FcwAebJa_Step_sys/ALG_AEBSystem/ALG_AEBController/AEB_LongTgtInfoCalc/LongObjCalcInfoCalc/AEBS_LongCollisionAssesApp/LongCollisionAvoidAssess/HighLvlBrkCalc/HighLvlBrkCalc4Warning/accelAvoidHighLvlBrkSituation1/accelAvoidLowLvlBrkCalc2 ==
  vx : Inport
  axAbsObj : Inport  Port=2
  vxAbsObj : Inport  Port=3
  subjVel : Inport  Port=4
  tObjStop : Inport  Port=5
  dxEgoBrake : Inport  Port=6
  AccelAvoidSituationJudge : Stateflow chart  TreatAsAtomicUnit=on
  Bus Creator : BusCreator  Inputs=6
  Bus Selector : BusSelector  OutputSignals=vxRelTargetObjCalc,axAbsTargetObjCalc
  Bus Selector1 : BusSelector  OutputSignals=dxEgoBrake,vxRelTargetObjCalc,tObjStop,subjVel,vxAbsTargetObjCalc,axAbsTargetObjCalc
  Bus Selector2 : BusSelector  OutputSignals=axAbsTargetObjCalc,vxRelTargetObjCalc,dxEgoBrake
  Bus Selector3 : BusSelector  OutputSignals=subjVel,vxAbsTargetObjCalc,axAbsTargetObjCalc,dxEgoBrake
  Merge : Merge  Inputs=4
  Situation1 : Subsystem  TreatAsAtomicUnit=on
  Situation2 : Subsystem  TreatAsAtomicUnit=on
  Situation3 : Subsystem  TreatAsAtomicUnit=on
  Situation4 : Subsystem  TreatAsAtomicUnit=on
  accelAvoidLowLvlBrkLimit : Subsystem
  accelAvoidLowLvlBrk : Outport
  wires:
  Bus Selector1/6 -> Situation1/6(axAbsObj)  "<axAbsTargetObjCalc>"
  Bus Selector1/5 -> Situation1/5(vxAbsObj)  "<vxAbsTargetObjCalc>"
  Bus Selector1/4 -> Situation1/4(subjVel)  "<subjVel>"
  Bus Selector1/3 -> Situation1/3(tObjStop)  "<tObjStop>"
  Bus Selector1/2 -> Situation1/2(vx)  "<vxRelTargetObjCalc>"
  Bus Selector1/1 -> Situation1/1(dxEgoBrake)  "<dxEgoBrake>"
  AccelAvoidSituationJudge/1(Situation1()) -> Situation1/Trigger
  Situation1/1(accelAvoidLowLvlBrk) -> Merge/1
  Situation2/1(accelAvoidLowLvlBrk) -> Merge/2
  Situation3/1(accelAvoidLowLvlBrk) -> Merge/3
  Situation4/1(accelAvoidLowLvlBrk) -> Merge/4
  AccelAvoidSituationJudge/2(Situation2()) -> [Situation2]
  AccelAvoidSituationJudge/3(Situation3()) -> [Situation3]
  AccelAvoidSituationJudge/4(Situation4()) -> [Situation4]
  [Situation2] -> Situation2/Trigger
  [Situation3] -> Situation3/Trigger
  [Situation4] -> Situation4/Trigger
  vxAbsObj/1 -> Bus Creator/3  "vxAbsTargetObjCalc"
  subjVel/1 -> Bus Creator/4  "subjVel"
  dxEgoBrake/1 -> Bus Creator/6  "dxEgoBrake"
  vx/1 -> Bus Creator/1  "vxRelTargetObjCalc"
  axAbsObj/1 -> Bus Creator/2  "axAbsTargetObjCalc"
  tObjStop/1 -> Bus Creator/5  "tObjStop"
  Bus Creator/1 -> Bus Selector3/1, Bus Selector2/1, Bus Selector1/1, Bus Selector/1
  Bus Selector/1 -> AccelAvoidSituationJudge/1(vxRelTargetObjCalc)  "<vxRelTargetObjCalc>"
  Bus Selector/2 -> AccelAvoidSituationJudge/2(axAbsTargetObjCalc)  "<axAbsTargetObjCalc>"
  Bus Selector2/3 -> Situation2/3(dxEgoBrake)  "<dxEgoBrake>"
  Bus Selector2/2 -> Situation2/2(vx)  "<vxRelTargetObjCalc>"
  Bus Selector2/1 -> Situation2/1(axAbsObj)  "<axAbsTargetObjCalc>"
  Bus Selector3/1 -> Situation3/1(subjVel)  "<subjVel>"
  Bus Selector3/2 -> Situation3/2(vxAbsObj)  "<vxAbsTargetObjCalc>"
  Bus Selector3/3 -> Situation3/3(axAbsObj)  "<axAbsTargetObjCalc>"
  Bus Selector3/4 -> Situation3/4(dxEgoBrake)  "<dxEgoBrake>"
  Merge/1 -> accelAvoidLowLvlBrkLimit/1(accelAvoidLowLvlBrkNoLimit)
  accelAvoidLowLvlBrkLimit/1(accelAvoidLowLvlBrk) -> accelAvoidLowLvlBrk/1

== system TosAeb/FcwAebJa_Step_sys/ALG_AEBSystem/ALG_AEBController/AEB_LongTgtInfoCalc/LongObjCalcInfoCalc/AEBS_LongCollisionAssesApp/LongCollisionAvoidAssess/HighLvlBrkCalc/HighLvlBrkCalc4Warning/accelAvoidHighLvlBrkSituation2/CollisionJuge ==
  tReaction : Inport
  TTC : Inport  Port=2
  Relational Operator : RelationalOperator  Operator=>  OutDataTypeStr=boolean  RndMeth=Simplest
  collisonFlg : Outport
  wires:
  tReaction/1 -> Relational Operator/1
  Relational Operator/1 -> collisonFlg/1
  TTC/1 -> Relational Operator/2

== system TosAeb/FcwAebJa_Step_sys/ALG_AEBSystem/ALG_AEBController/AEB_LongTgtInfoCalc/LongObjCalcInfoCalc/AEBS_LongCollisionAssesApp/LongCollisionAvoidAssess/HighLvlBrkCalc/HighLvlBrkCalc4Warning/accelAvoidHighLvlBrkSituation2/CollisonOcurred ==
  Action Port : ActionPort  ActionPortLabel=if(u1)
  Constant : Constant  Value=CNPEBSAccelNegInf
  accelAvoidHighLvlBrk : Outport
  wires:
  Constant/1 -> accelAvoidHighLvlBrk/1  "CNPEBSAccelNegInf"

== system TosAeb/FcwAebJa_Step_sys/ALG_AEBSystem/ALG_AEBController/AEB_LongTgtInfoCalc/LongObjCalcInfoCalc/AEBS_LongCollisionAssesApp/LongCollisionAvoidAssess/HighLvlBrkCalc/HighLvlBrkCalc4Warning/accelAvoidHighLvlBrkSituation2/accelAvoidHighLvlBrkCalc ==
  dxFrontBumper : Inport
  vx : Inport  Port=2
  tReaction : Inport  Port=3
  ax : Inport  Port=4
  subjLongAccel : Inport  Port=5
  subjVel : Inport  Port=6
  axAbsObj : Inport  Port=7
  Action Port : ActionPort  ActionPortLabel=else
  If : If  IfExpression=u1 <= 0
  If Action Subsystem : Subsystem  TreatAsAtomicUnit=on
  If Action Subsystem1 : Subsystem  TreatAsAtomicUnit=on
  Merge : Merge
  MovingSateCalcAfterReactionTime : Subsystem
  tObjStopCalc : Subsystem  TreatAsAtomicUnit=on
  accelAvoidHighLvlBrk : Outport
  wires:
  dxFrontBumper/1 -> MovingSateCalcAfterReactionTime/1(dxFrontBumper)
  [dxRelTargetPredict] -> If Action Subsystem1/6(dxEgoBrake)
  [subjVelPredict] -> If Action Subsystem1/4(subjVel)
  MovingSateCalcAfterReactionTime/4(subjVelPredict) -> [subjVelPredict]
  MovingSateCalcAfterReactionTime/2(vxPredict) -> If Action Subsystem1/1(vx)
  axAbsObj/1 -> If Action Subsystem1/2(axAbsObj), tObjStopCalc/1(accelRel)
  MovingSateCalcAfterReactionTime/3(vxAbsObjPredict) -> If Action Subsystem1/3(vxAbsObj), tObjStopCalc/2(velRel)
  If Action Subsystem/1(accelAvoidHighLvlBrk) -> Merge/1
  If Action Subsystem1/1(accelAvoidHighLvlBrk) -> Merge/2
  If/2 -> If Action Subsystem1/Action
  If/1 -> If Action Subsystem/Action
  MovingSateCalcAfterReactionTime/1(dxFrontBumperPredict) -> If/1, [dxRelTargetPredict]
  subjLongAccel/1 -> MovingSateCalcAfterReactionTime/5(subjLongAccel)
  subjVel/1 -> MovingSateCalcAfterReactionTime/6(subjVel)
  ax/1 -> MovingSateCalcAfterReactionTime/4(ax)
  tReaction/1 -> MovingSateCalcAfterReactionTime/3(tReaction)
  vx/1 -> MovingSateCalcAfterReactionTime/2(vx)
  Merge/1 -> accelAvoidHighLvlBrk/1
  tObjStopCalc/1(tStop) -> If Action Subsystem1/5(tObjStop)

== system TosAeb/FcwAebJa_Step_sys/ALG_AEBSystem/ALG_AEBController/AEB_LongTgtInfoCalc/LongObjCalcInfoCalc/AEBS_LongCollisionAssesApp/LongCollisionAvoidAssess/HighLvlBrkCalc/HighLvlBrkCalc4Warning/accelAvoidHighLvlBrkSituation3/CollisionJuge ==
  tReaction : Inport
  TTC : Inport  Port=2
  Relational Operator : RelationalOperator  Operator=>  OutDataTypeStr=boolean  RndMeth=Simplest
  collisonFlg : Outport
  wires:
  tReaction/1 -> Relational Operator/1
  Relational Operator/1 -> collisonFlg/1
  TTC/1 -> Relational Operator/2

== system TosAeb/FcwAebJa_Step_sys/ALG_AEBSystem/ALG_AEBController/AEB_LongTgtInfoCalc/LongObjCalcInfoCalc/AEBS_LongCollisionAssesApp/LongCollisionAvoidAssess/HighLvlBrkCalc/HighLvlBrkCalc4Warning/accelAvoidHighLvlBrkSituation3/If Action Subsystem ==
  Action Port : ActionPort  ActionPortLabel=if(u1)
  Constant : Constant  Value=CNaccelAvoidMin
  accelAvoidHighLvlBrk : Outport
  wires:
  Constant/1 -> accelAvoidHighLvlBrk/1  "CNPEBSAccelNegInf"

== system TosAeb/FcwAebJa_Step_sys/ALG_AEBSystem/ALG_AEBController/AEB_LongTgtInfoCalc/LongObjCalcInfoCalc/AEBS_LongCollisionAssesApp/LongCollisionAvoidAssess/HighLvlBrkCalc/HighLvlBrkCalc4Warning/accelAvoidHighLvlBrkSituation3/If Action Subsystem1 ==
  subjLongAccel : Inport
  tReaction : Inport  Port=2
  subjVel : Inport  Port=3
  axAbsObj : Inport  Port=4
  vxAbsObj : Inport  Port=5
  dxFrontBumper : Inport  Port=6
  Action Port : ActionPort  ActionPortLabel=else
  CollisionOcurredCase : Subsystem  TreatAsAtomicUnit=on
  DistReactionCalc : Subsystem
  If : If  IfExpression=u1
  Merge : Merge
  accelAvoidHighLvlBrkCalc : Subsystem  TreatAsAtomicUnit=on
  accelAvoidHighLvlBrk : Outport
  wires:
  accelAvoidHighLvlBrkCalc/1(accelAvoidHighLvlBrk) -> Merge/2
  [subjVel] -> accelAvoidHighLvlBrkCalc/3(subjVel)
  [tReaction] -> accelAvoidHighLvlBrkCalc/2(tReaction)
  [subjAccel] -> accelAvoidHighLvlBrkCalc/1(subjLongAccel)
  DistReactionCalc/2(dxRelTargetPredict) -> accelAvoidHighLvlBrkCalc/4(dxEgoBrake)
  CollisionOcurredCase/1(accelAvoidHighLvlBrk) -> Merge/1
  If/2 -> accelAvoidHighLvlBrkCalc/Action
  If/1 -> CollisionOcurredCase/Action
  DistReactionCalc/1(collisionOcurredFlag) -> If/1
  dxFrontBumper/1 -> DistReactionCalc/6(dxFrontBumper)
  Merge/1 -> accelAvoidHighLvlBrk/1
  vxAbsObj/1 -> DistReactionCalc/5(vxAbsObj)
  axAbsObj/1 -> DistReactionCalc/4(axAbsObj)
  subjVel/1 -> DistReactionCalc/3(subjVel), [subjVel]
  tReaction/1 -> DistReactionCalc/2(tReaction), [tReaction]
  subjLongAccel/1 -> DistReactionCalc/1(subjLongAccel), [subjAccel]

== system TosAeb/FcwAebJa_Step_sys/ALG_AEBSystem/ALG_AEBController/AEB_LongTgtInfoCalc/LongObjCalcInfoCalc/AEBS_LongCollisionAssesApp/LongCollisionAvoidAssess/HighLvlBrkCalc/HighLvlBrkCalc4Warning/accelAvoidHighLvlBrkSituation4/accelAvoidHighLvlBrkCalc ==
  tReaction : Inport
  TTC : Inport  Port=2
  Constant : Constant  Value=CNaccelAvoidMax
  Constant1 : Constant  Value=CNaccelAvoidMin
  Relational Operator : RelationalOperator  Operator=<=  OutDataTypeStr=boolean  RndMeth=Simplest
  Switch : Switch  Criteria=u2 ~= 0
  accelAvoidHighLvlBrk : Outport
  wires:
  Switch/1 -> accelAvoidHighLvlBrk/1
  Constant1/1 -> Switch/3
  Constant/1 -> Switch/1
  TTC/1 -> Relational Operator/2
  Relational Operator/1 -> Switch/2
  tReaction/1 -> Relational Operator/1

== system TosAeb/FcwAebJa_Step_sys/ALG_AEBSystem/ALG_AEBController/AEB_LongTgtInfoCalc/LongObjCalcInfoCalc/AEBS_LongCollisionAssesApp/LongCollisionAvoidAssess/HighLvlBrkCalc/HighLvlBrkCalc4Warning/accelAvoidHighLvlBrkSituation5/If Action Subsystem ==
  tReaction : Inport
  TTC : Inport  Port=2
  Action Port : ActionPort  ActionPortLabel=if(u1 <= u2)
  Constant : Constant  Value=CNaccelAvoidMax
  Constant1 : Constant  Value=CNaccelAvoidMin
  Relational Operator : RelationalOperator  Operator=<=  OutDataTypeStr=boolean  RndMeth=Simplest
  Switch : Switch  Criteria=u2 > Threshold
  accelAvoidHighLvlBrk : Outport
  wires:
  Switch/1 -> accelAvoidHighLvlBrk/1
  Constant1/1 -> Switch/3
  Constant/1 -> Switch/1
  Relational Operator/1 -> Switch/2
  TTC/1 -> Relational Operator/2
  tReaction/1 -> Relational Operator/1

== system TosAeb/FcwAebJa_Step_sys/ALG_AEBSystem/ALG_AEBController/AEB_LongTgtInfoCalc/LongObjCalcInfoCalc/AEBS_LongCollisionAssesApp/LongCollisionAvoidAssess/HighLvlBrkCalc/HighLvlBrkCalc4Warning/accelAvoidHighLvlBrkSituation5/If Action Subsystem1 ==
  tReaction : Inport
  TTC : Inport  Port=2
  subjLongAccel : Inport  Port=3
  tEgoStop : Inport  Port=4
  subjVel : Inport  Port=5
  axAbsObj : Inport  Port=6
  dxFrontBumper : Inport  Port=7
  vxAbsObj : Inport  Port=8
  Action Port : ActionPort  ActionPortLabel=else
  CollisionJuge : Subsystem
  If : If  IfExpression=u1
  If Action Subsystem : Subsystem  TreatAsAtomicUnit=on
  If Action Subsystem1 : Subsystem  TreatAsAtomicUnit=on
  Merge : Merge
  accelAvoidHighLvlBrk : Outport
  wires:
  dxFrontBumper/1 -> If Action Subsystem1/6(dxFrontBumper)
  vxAbsObj/1 -> If Action Subsystem1/5(vxAbsObj)
  axAbsObj/1 -> If Action Subsystem1/4(axAbsObj)
  subjVel/1 -> If Action Subsystem1/3(subjVel)
  subjLongAccel/1 -> If Action Subsystem1/1(subjLongAccel)
  tReaction/1 -> CollisionJuge/1(tReaction)
  Merge/1 -> accelAvoidHighLvlBrk/1
  If Action Subsystem1/1(accelAvoidHighLvlBrk) -> Merge/2
  If Action Subsystem/1(accelAvoidHighLvlBrk) -> Merge/1
  If/2 -> If Action Subsystem1/Action
  If/1 -> If Action Subsystem/Action
  TTC/1 -> CollisionJuge/2(TTC)
  CollisionJuge/1(collisonFlg) -> If/1
  tEgoStop/1 -> If Action Subsystem1/2(tEgoStop)

== system TosAeb/FcwAebJa_Step_sys/ALG_AEBSystem/ALG_AEBController/AEB_LongTgtInfoCalc/LongObjCalcInfoCalc/AEBS_LongCollisionAssesApp/LongCollisionAvoidAssess/LowLvlBrkCalc/accelAvoidLowLvlBrkCalc/Situation1/Case1 ==
  axAbsObj : Inport
  vx : Inport  Port=2
  dxEgoBrake : Inport  Port=3
  Action Port : ActionPort  ActionPortLabel=if(u1)
  Divide : Product  Inputs=*/
  Gain : Gain  Gain=2
  Math Function : Math  Operator=square  SignedPower=on  SaturateOnIntegerOverflow=on
  Subtract : Sum  Inputs=+-
  accelAvoid : Outport
  wires:
  Subtract/1 -> accelAvoid/1
  axAbsObj/1 -> Subtract/1
  Divide/1 -> Subtract/2
  dxEgoBrake/1 -> Gain/1
  Gain/1 -> Divide/2
  Math Function/1 -> Divide/1
  vx/1 -> Math Function/1

== system TosAeb/FcwAebJa_Step_sys/ALG_AEBSystem/ALG_AEBController/AEB_LongTgtInfoCalc/LongObjCalcInfoCalc/AEBS_LongCollisionAssesApp/LongCollisionAvoidAssess/LowLvlBrkCalc/accelAvoidLowLvlBrkCalc/Situation1/Case2 ==
  note: vEgo^2
      | aAvoid = ----------------------------------
      |                   vObj^2
      |                  ---------  - 2(dRel - dScure)
      |                   aObj
  subjVel : Inport
  vxAbsObj : Inport  Port=2
  axAbsObj : Inport  Port=3
  dxEgoBrake : Inport  Port=4
  Action Port : ActionPort  ActionPortLabel=else
  Divide : Product  Inputs=*/
  Divide1 : Product  Inputs=*/
  Gain : Gain  Gain=2
  Math Function : Math  Operator=square  SignedPower=on  SaturateOnIntegerOverflow=on
  Math Function1 : Math  Operator=square  SignedPower=on  SaturateOnIntegerOverflow=on
  Subtract : Sum  Inputs=+-
  accelAvoid : Outport
  wires:
  Divide1/1 -> accelAvoid/1
  Math Function1/1 -> Divide1/1
  Subtract/1 -> Divide1/2
  subjVel/1 -> Math Function1/1
  Gain/1 -> Subtract/2
  dxEgoBrake/1 -> Gain/1
  Divide/1 -> Subtract/1
  axAbsObj/1 -> Divide/2
  Math Function/1 -> Divide/1
  vxAbsObj/1 -> Math Function/1

== system TosAeb/FcwAebJa_Step_sys/ALG_AEBSystem/ALG_AEBController/AEB_LongTgtInfoCalc/LongObjCalcInfoCalc/AEBS_LongCollisionAssesApp/LongCollisionAvoidAssess/LowLvlBrkCalc/accelAvoidLowLvlBrkCalc/Situation1/collisionFirstFlgCalc ==
  note: Calculate Ego and Obj Collision Time and
      |  Determine if the Collision Time is less than the object stop time
  dxEgoBrake : Inport
  vx : Inport  Port=2
  tObjStop : Inport  Port=3
  CNTTCMAX : Constant  Value=CNTTCMAX
  Divide : Product  Inputs=*/
  Gain : Gain  Gain=-1
  Gain1 : Gain  Gain=2
  MinMax : MinMax  Inputs=2
  Relational Operator : RelationalOperator  Operator=<  OutDataTypeStr=boolean  RndMeth=Simplest
  collisionFirstFlg : Outport
  wires:
  CNTTCMAX/1 -> MinMax/2
  MinMax/1 -> Relational Operator/1
  Relational Operator/1 -> collisionFirstFlg/1  "collisionFirstFlg"
  tObjStop/1 -> Relational Operator/2
  Gain1/1 -> MinMax/1
  Gain/1 -> Divide/2
  vx/1 -> Gain/1
  dxEgoBrake/1 -> Divide/1
  Divide/1 -> Gain1/1

== system TosAeb/FcwAebJa_Step_sys/ALG_AEBSystem/ALG_AEBController/AEB_LongTgtInfoCalc/LongObjCalcInfoCalc/AEBS_LongCollisionAssesApp/TTCCalc/TTCCalc/Situation2/SloveTTCQuadraticEquation/AccelRelEqualsZero ==
  distRel : Inport
  velRel : Inport  Port=2
  AccelRelEqualZero : ActionPort  ActionPortLabel=if(u1)
  Chart : Stateflow chart  TreatAsAtomicUnit=on
  TTC : Outport
  wires:
  Chart/1(TTC) -> TTC/1  "TTC"
  distRel/1 -> Chart/1(distRel)  "distRel"
  velRel/1 -> Chart/2(velRel)  "velRel"

== system TosAeb/FcwAebJa_Step_sys/ALG_AEBSystem/ALG_AEBController/AEB_LongTgtInfoCalc/LongObjCalcInfoCalc/AEBS_LongCollisionAssesApp/TTCCalc/TTCCalc/Situation2/SloveTTCQuadraticEquation/AccelRelUnequalZero ==
  accelRel : Inport
  velRel : Inport  Port=2
  distRel : Inport  Port=3
  AccelRelUnequalZero : ActionPort  ActionPortLabel=else
  DeltaCalcu : Subsystem
  DeltaGreaterEqualZero : Subsystem  TreatAsAtomicUnit=on
  DeltaSmallerZero : Subsystem  TreatAsAtomicUnit=on
  JudeDelta : If  IfExpression=u1 >= 0
  Merge : Merge
  TTC : Outport
  wires:
  Merge/1 -> TTC/1  "TTC"
  DeltaCalcu/1(delta) -> JudeDelta/1, DeltaGreaterEqualZero/1(delta)  "delta"
  accelRel/1 -> DeltaCalcu/1(accelRel), DeltaGreaterEqualZero/3(accelRel)  "accelRel"
  velRel/1 -> DeltaCalcu/2(velRel), DeltaGreaterEqualZero/2(velRel)  "velRel"
  distRel/1 -> DeltaCalcu/3(distRel)  "distRel"
  DeltaSmallerZero/1(TTC) -> Merge/2
  DeltaGreaterEqualZero/1(TTC) -> Merge/1
  JudeDelta/1 -> DeltaGreaterEqualZero/Action
  JudeDelta/2 -> [DeltaSmallZero]
  [DeltaSmallZero] -> DeltaSmallerZero/Action

== system TosAeb/FcwAebJa_Step_sys/ALG_AEBSystem/ALG_AEBController/AEB_LongTgtInfoCalc/LongObjCalcInfoCalc/AEBS_LongCollisionAssesApp/TTCCalc/TTCCalc/Situation2/SloveTTCQuadraticEquation/accelZeroFlgCalc ==
  accel : Inport
  Abs : Abs
  P_LongCA_AccelObjFiltThesTTC : simulink/Logic and Bit Operations/Compare To Constant  relop=<=  const=P_AEB_LongCA_AccelObjFiltThesTTC  OutDataTypeStr=boolean
  accelZeroFlg : Outport
  wires:
  Abs/1 -> P_LongCA_AccelObjFiltThesTTC/1
  P_LongCA_AccelObjFiltThesTTC/1 -> accelZeroFlg/1  "accelZeroFlg"
  accel/1 -> Abs/1  "accel"
